simulink model "FaultSimulator"  (R2022a)
settings: Solver=FixedStepAuto  StartTime=0.0  StopTime=10.0  FixedStep=1e-3  RelTol=1e-3
values from neighbouring files (symbols this model uses):
  A = A_set(i)    [DatacreatorForChapter3.m]    (also in parameterSetting.m = 0.0005)
  base = Mb*9.8/4+mw*9.8+0.5*9.8*Mv    (= 46305)    [parameterSetting.m]
  d = 2.6    [parameterSetting.m]
  I = 3055e-8    (= 3.055e-05)    [parameterSetting.m]
  Jv = 3044    [parameterSetting.m]
  kf = 6.67e7    (= 66700000)    [parameterSetting.m]
  Kv = 1.5e6    (= 1500000)    [parameterSetting.m]
  L = beamLength(k)*210    [BuildDataSample.m]    (also in Figure3_1.m = 210; parameterSetting.m = 210)
  Ln = length_set(i)    [DatacreatorForChapter3.m]
  Ln2 = 0    [DatacreatorForChapter3.m]
  m = 7800*(7684e-6)    (= 59.9352)    [parameterSetting.m]
  Mv = 4060/2    (= 2030)    [parameterSetting.m]
  mw = 1670/2    (= 835)    [parameterSetting.m]
  N1 = 0    [DatacreatorForChapter3.m]
  N2 = 0    [DatacreatorForChapter3.m]
  position = 0.55*L    [DatacreatorForChapter3.m]    (also in Figure3_3ToFigure3_8andTable4_1.m = 115.5; Figure3_8.m = 115.5; Figure3_9.m = 105; parameterSetting.m = 105)
  r = 0.25    [Figure3_3ToFigure3_8andTable4_1.m]    (also in parameterSetting.m = 0.42)
  R = 0.42    [parameterSetting.m]
  v = vkmh/3.6    [BuildDataSample.m]    (also in DatacreatorForChapter3.m = vkmh_set(j)/3.6; Figure3_1.m = vkmh_set(i)/3.6; Figure3_3ToFigure3_8andTable4_1.m = 13.8888888889; Figure3_8.m = 50)
  vkmh = vkmh_set(j)    [BuildDataSample.m]    (also in parameterSetting.m = 100)
(part 1 of 157)
settings Model: Creator=Spicydel  LastModifiedBy=Spicydel

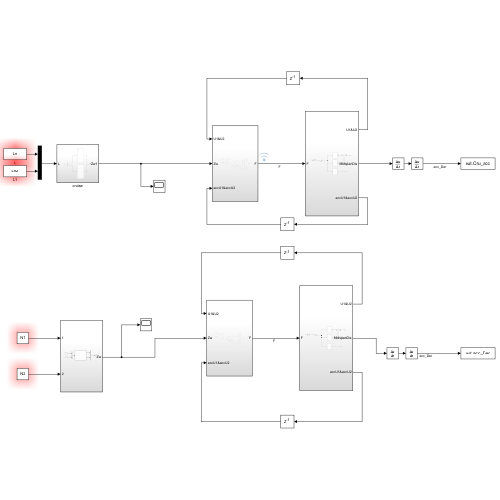
[thumbnail: Simulink's stored preview of the top-level diagram]
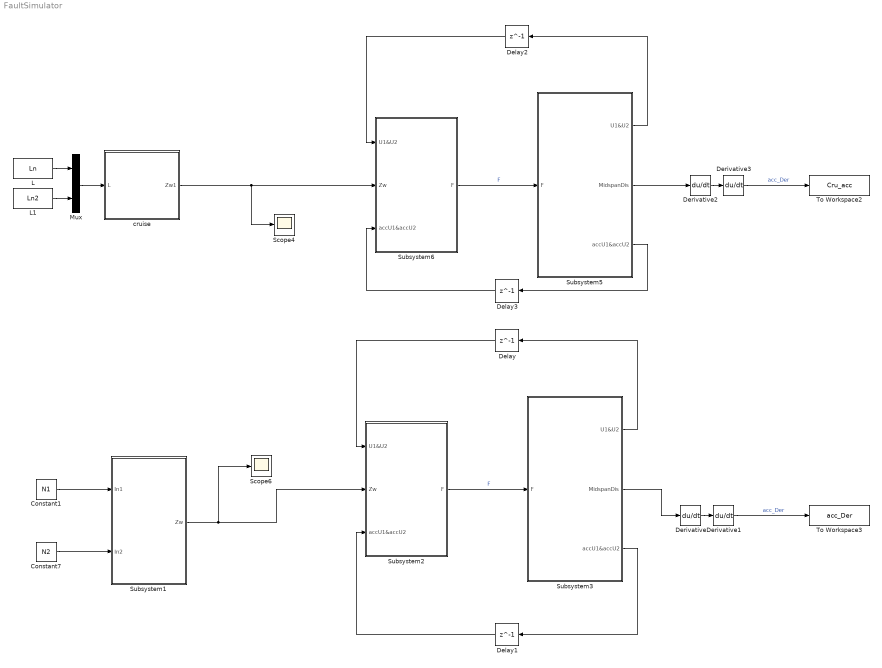
== system FaultSimulator ==
  Constant1 : Constant  Value=N1
  Constant7 : Constant  Value=N2
  Delay{,1..3} (4 alike) : Delay  DelayLength=1
  Derivative{,1..3} (4 alike) : Derivative
  L : Constant  Value=Ln
  L1 : Constant  Value=Ln2
  Mux : Mux  Inputs=2
  Scope4 : Scope
  Scope6 : Scope
  Subsystem1 : Subsystem
  Subsystem2 : Subsystem
  Subsystem3 : Subsystem
  Subsystem5 : Subsystem
  Subsystem6 : Subsystem
  To Workspace2 : ToWorkspace  VariableName=Cru_acc  SaveFormat=Timeseries  SampleTime=-1
  To Workspace3 : ToWorkspace  VariableName=acc_Der  SaveFormat=Timeseries  SampleTime=-1
  cruise : Subsystem
  wires:
  Subsystem5/2(MidspanDis) -> Derivative2/1
  Delay2/1 -> Subsystem6/1(U1&U2)
  Subsystem5/1(U1&U2) -> Delay2/1
  L/1 -> Mux/1
  Delay3/1 -> Subsystem6/3(accU1&accU2)
  Subsystem6/1(F) -> Subsystem5/1(F)  "F"
  cruise/1(Zw1) -> Scope4/1, Subsystem6/2(Zw)
  Derivative3/1 -> To Workspace2/1  "acc_Der"
  Derivative2/1 -> Derivative3/1
  Subsystem5/3(accU1&accU2) -> Delay3/1
  L1/1 -> Mux/2
  Mux/1 -> cruise/1(L)
  Subsystem3/1(U1&U2) -> Delay/1
  Derivative1/1 -> To Workspace3/1  "acc_Der"
  Constant1/1 -> Subsystem1/1(In1)
  Constant7/1 -> Subsystem1/2(In2)
  Subsystem3/2(MidspanDis) -> Derivative/1
  Delay/1 -> Subsystem2/1(U1&U2)
  Subsystem1/1(Zw) -> Scope6/1, Subsystem2/2(Zw)
  Subsystem2/1(F) -> Subsystem3/1(F)  "F"
  Subsystem3/3(accU1&accU2) -> Delay1/1
  Delay1/1 -> Subsystem2/3(accU1&accU2)
  Derivative/1 -> Derivative1/1

== system FaultSimulator/Subsystem1 ==
  In1 : Inport
  In2 : Inport  Port=2
  Mux1 : Mux  Inputs=2
  Mux2 : Mux  Inputs=2
  poly : Subsystem
  Zw : Outport
  wires:
  poly/1(wheel1) -> Mux2/1
  In2/1 -> Mux1/2
  poly/2(wheel2) -> Mux2/2
  Mux1/1 -> poly/1(N)
  In1/1 -> Mux1/1
  Mux2/1 -> Zw/1

== system FaultSimulator/Subsystem2 ==
  U1&U2 : Inport
  Zw : Inport  Port=2
  accU1&accU2 : Inport  Port=3
  Add : Sum  Inputs=+---
  Add1 : Sum  Inputs=-+
  Add2 : Sum  Inputs=--++-
  Add3 : Sum  Inputs=-++--
  Add4 : Sum
  Add5 : Sum  Inputs=-+
  Add6 : Sum  Inputs=-+
  Add7 : Sum  Inputs=++--
  Constant : Constant  Value=base
  Demux{,1..6} (7 alike) : Demux  Outputs=2
  Derivative{,1..3} (4 alike) : Derivative
  Gain : Gain  Gain=2*Kv/Mv
  Gain1 : Gain  Gain=Kv*d*d/Jv/2
  Gain12 : Gain  Gain=Kv/Mv
  Gain14 : Gain  Gain=Kv*d/Jv/2
  Gain2 : Gain  Gain=Kv
  Gain3 : Gain  Gain=Kv/Mv
  Gain4 : Gain  Gain=Kv*d/Jv/2
  Gain5 : Gain  Gain=d*Kv/2
  Gain7 : Gain  Gain=mw
  Gain8 : Gain  Gain=mw
  Gain9 : Gain  Gain=Kv
  Integrator{,1..3} (4 alike) : Integrator
  Mux : Mux  Inputs=2
  F : Outport
  wires:
  Integrator/1 -> Integrator1/1
  Integrator1/1 -> Gain2/1, Gain/1  "Zv"
  Add2/1 -> Integrator/1  "Z_dot_dot"
  Integrator2/1 -> Integrator3/1
  Integrator3/1 -> Gain5/1, Gain1/1  "theta"
  Add3/1 -> Integrator2/1  "theta_dot_dot"
  Gain5/1 -> Add/2, Add7/2
  Zw/1 -> [Zw]
  Gain/1 -> Add2/5  "Z_dot_dot"
  Demux/1 -> Add2/3
  Demux/2 -> Add2/4
  Gain1/1 -> Add3/5  "theta_dot_dot"
  Demux2/1 -> Add3/1
  Demux2/2 -> Add3/2
  U1&U2/1 -> [U1u2]
  Add4/1 -> F/1
  Constant/1 -> Add4/2
  [U1u2] -> Gain12/1
  Gain12/1 -> Demux1/1
  Demux1/2 -> Add2/2
  Demux1/1 -> Add2/1
  [U1u2] -> Gain14/1
  Gain14/1 -> Demux3/1
  Demux3/1 -> Add3/3
  Demux3/2 -> Add3/4
  Gain2/1 -> Add7/1, Add/1
  Mux/1 -> Add4/1
  [Zw] -> Gain3/1
  Gain3/1 -> Demux/1
  Gain4/1 -> Demux2/1
  [Zw] -> Gain4/1
  [U1u2] -> Add1/1
  [Zw] -> Demux6/1, Add1/2
  Add1/1 -> Gain9/1
  Derivative/1 -> Derivative1/1
  accU1&accU2/1 -> Demux5/1
  Demux6/1 -> Derivative/1
  Derivative2/1 -> Derivative3/1
  Demux6/2 -> Derivative2/1
  Derivative1/1 -> Add5/2
  Demux5/1 -> Add5/1
  Derivative3/1 -> Add6/2
  Demux5/2 -> Add6/1
  Demux4/1 -> Add/3
  Add5/1 -> Gain7/1
  Add6/1 -> Gain8/1
  Gain7/1 -> Add/4
  Gain9/1 -> Demux4/1
  Demux4/2 -> Add7/3
  Gain8/1 -> Add7/4
  Add7/1 -> Mux/2  "F2"
  Add/1 -> Mux/1  "F1"

== system FaultSimulator/Subsystem3 ==
  F : Inport
  Add : Sum
  Demux1 : Demux  Outputs=2
  Demux2 : Demux  Outputs=2
  Demux3 : Demux  Outputs=2
  Gain1 : Gain  Gain=-1
  Gain2 : Gain  Gain=-1
  Mux : Mux  Inputs=2
  Mux1 : Mux  Inputs=2
  Mux2 : Mux  Inputs=2
  Subsystem : Subsystem
  Subsystem1 : Subsystem
  Subsystem3 : Subsystem
  U1&U2 : Outport
  MidspanDis : Outport  Port=2
  accU1&accU2 : Outport  Port=3
  wires:
  Subsystem/1(u1) -> Gain1/1
  Subsystem1/1(u2) -> Gain2/1
  Subsystem3/1(Midspan) -> MidspanDis/1
  Gain1/1 -> Demux2/1
  F/1 -> Demux1/1
  Demux1/1 -> Add/1
  Demux1/2 -> Add/2
  Add/1 -> Mux1/2, Mux1/1
  Mux1/1 -> Subsystem3/1(F), Subsystem1/1(F), Subsystem/1(F)
  Gain2/1 -> Demux3/1
  Demux2/1 -> Mux/1
  Demux3/1 -> Mux/2
  Mux2/1 -> accU1&accU2/1
  Mux/1 -> U1&U2/1
  Demux2/2 -> Mux2/1
  Demux3/2 -> Mux2/2

== system FaultSimulator/Subsystem5 ==
  F : Inport
  Add : Sum
  Demux1 : Demux  Outputs=2
  Demux2 : Demux  Outputs=2
  Demux3 : Demux  Outputs=2
  Gain1 : Gain  Gain=-1
  Gain2 : Gain  Gain=-1
  Mux : Mux  Inputs=2
  Mux1 : Mux  Inputs=2
  Mux2 : Mux  Inputs=2
  Subsystem : Subsystem
  Subsystem1 : Subsystem
  Subsystem3 : Subsystem
  U1&U2 : Outport
  MidspanDis : Outport  Port=2
  accU1&accU2 : Outport  Port=3
  wires:
  Demux3/2 -> Mux2/2
  Demux2/2 -> Mux2/1
  Mux/1 -> U1&U2/1
  Mux2/1 -> accU1&accU2/1
  Demux3/1 -> Mux/2
  Demux2/1 -> Mux/1
  Gain2/1 -> Demux3/1
  Mux1/1 -> Subsystem/1(F), Subsystem1/1(F), Subsystem3/1(F)
  Add/1 -> Mux1/1, Mux1/2
  Demux1/2 -> Add/2
  Demux1/1 -> Add/1
  F/1 -> Demux1/1
  Gain1/1 -> Demux2/1
  Subsystem1/1(u2) -> Gain2/1
  Subsystem/1(u1) -> Gain1/1
  Subsystem3/1(Midspan) -> MidspanDis/1

== system FaultSimulator/Subsystem6 ==
  U1&U2 : Inport
  Zw : Inport  Port=2
  accU1&accU2 : Inport  Port=3
  Add : Sum  Inputs=+---
  Add1 : Sum  Inputs=-+
  Add2 : Sum  Inputs=--++-
  Add3 : Sum  Inputs=-++--
  Add4 : Sum
  Add5 : Sum  Inputs=-+
  Add6 : Sum  Inputs=-+
  Add7 : Sum  Inputs=++--
  Constant : Constant  Value=base
  Demux{,1..6} (7 alike) : Demux  Outputs=2
  Derivative{,1..3} (4 alike) : Derivative
  Gain : Gain  Gain=2*Kv/Mv
  Gain1 : Gain  Gain=Kv*d*d/Jv/2
  Gain12 : Gain  Gain=Kv/Mv
  Gain14 : Gain  Gain=Kv*d/Jv/2
  Gain2 : Gain  Gain=Kv
  Gain3 : Gain  Gain=Kv/Mv
  Gain4 : Gain  Gain=Kv*d/Jv/2
  Gain5 : Gain  Gain=d*Kv/2
  Gain7 : Gain  Gain=mw
  Gain8 : Gain  Gain=mw
  Gain9 : Gain  Gain=Kv
  Integrator{,1..3} (4 alike) : Integrator
  Mux : Mux  Inputs=2
  Scope : Scope
  Scope1 : Scope
  Scope2 : Scope
  F : Outport
  wires:
  Add/1 -> Scope1/1, Mux/1  "F1"
  Add7/1 -> Mux/2, Scope2/1  "F2"
  Gain8/1 -> Add7/4
  Demux4/2 -> Add7/3
  Gain9/1 -> Demux4/1
  Gain7/1 -> Add/4
  Add6/1 -> Gain8/1
  Add5/1 -> Gain7/1
  Demux4/1 -> Add/3
  Demux5/2 -> Add6/1
  Derivative3/1 -> Add6/2
  Demux5/1 -> Add5/1
  Derivative1/1 -> Add5/2
  Demux6/2 -> Derivative2/1
  Derivative2/1 -> Derivative3/1
  Demux6/1 -> Derivative/1
  accU1&accU2/1 -> Demux5/1
  Derivative/1 -> Derivative1/1
  Add1/1 -> Gain9/1
  [Zw] -> Add1/2, Demux6/1
  [U1u2] -> Add1/1
  [Zw] -> Gain4/1
  Gain4/1 -> Demux2/1
  Gain3/1 -> Demux/1
  [Zw] -> Gain3/1
  Mux/1 -> Add4/1
  Gain2/1 -> Add/1, Add7/1
  Demux3/2 -> Add3/4
  Demux3/1 -> Add3/3
  Gain14/1 -> Demux3/1
  [U1u2] -> Gain14/1
  Demux1/1 -> Add2/1
  Demux1/2 -> Add2/2
  Gain12/1 -> Demux1/1
  [U1u2] -> Gain12/1
  Constant/1 -> Add4/2
  Add4/1 -> Scope/1, F/1
  U1&U2/1 -> [U1u2]
  Demux2/2 -> Add3/2
  Demux2/1 -> Add3/1
  Gain1/1 -> Add3/5  "theta_dot_dot"
  Demux/2 -> Add2/4
  Demux/1 -> Add2/3
  Gain/1 -> Add2/5  "Z_dot_dot"
  Zw/1 -> [Zw]
  Gain5/1 -> Add7/2, Add/2
  Add3/1 -> Integrator2/1  "theta_dot_dot"
  Integrator3/1 -> Gain1/1, Gain5/1  "theta"
  Integrator2/1 -> Integrator3/1
  Add2/1 -> Integrator/1  "Z_dot_dot"
  Integrator1/1 -> Gain/1, Gain2/1  "Zv"
  Integrator/1 -> Integrator1/1

== system FaultSimulator/cruise ==
  L : Inport
  Demux : Demux  Outputs=2
  Mux : Mux  Inputs=2
  Subsystem1 : Subsystem
  Subsystem2 : Subsystem
  Zw1 : Outport
  wires:
  L/1 -> Demux/1
  Demux/1 -> Subsystem1/1(In1)
  Demux/2 -> Subsystem2/1(In1)
  Subsystem1/1(Output) -> Mux/1
  Subsystem2/1(Output) -> Mux/2
  Mux/1 -> Zw1/1

== system FaultSimulator/Subsystem1/poly ==
  N : Inport
  Clock : Clock
  Constant : Constant  Value=v
  Demux : Demux  Outputs=2
  Gain1 : Gain  Gain=1/r
  Product1 : Product
  Subsystem : Subsystem
  Subsystem1 : Subsystem
  wheel1 : Outport
  wheel2 : Outport  Port=2
  wires:
  Constant/1 -> Gain1/1
  Gain1/1 -> Product1/1
  Clock/1 -> Product1/2
  Product1/1 -> [omega]
  [omega] -> Subsystem/2(In2)
  Subsystem/1(Out1) -> wheel1/1
  [omega] -> Subsystem1/2(In2)
  Subsystem1/1(Out1) -> wheel2/1
  N/1 -> Demux/1
  Demux/1 -> Subsystem/1(In1)
  Demux/2 -> Subsystem1/1(In1)

== system FaultSimulator/Subsystem3/Subsystem ==
  F : Inport
  Add : Sum  Inputs=+++++++
  Constant1 : Constant  Value=21
  Constant11 : Constant
  Constant2 : Constant  Value=41
  Constant3 : Constant  Value=61
  Constant4 : Constant  Value=81
  Constant5 : Constant  Value=101
  Constant6 : Constant  Value=121
  Subsystem : Subsystem
  Subsystem1 : Subsystem
  Subsystem2 : Subsystem
  Subsystem3 : Subsystem
  Subsystem4 : Subsystem
  Subsystem5 : Subsystem
  Subsystem6 : Subsystem
  u1 : Outport
  wires:
  F/1 -> [Ff]
  Constant11/1 -> Subsystem/1(In1)
  [Ff] -> Subsystem/2(In2)
  [Ff] -> Subsystem1/2(In2)
  Constant1/1 -> Subsystem1/1(In1)
  Constant2/1 -> Subsystem2/1(In1)
  [Ff] -> Subsystem2/2(In2)
  [Ff] -> Subsystem3/2(In2)
  Constant3/1 -> Subsystem3/1(In1)
  Constant4/1 -> Subsystem4/1(In1)
  [Ff] -> Subsystem4/2(In2)
  Subsystem/1(Out1) -> Add/1
  Subsystem1/1(Out1) -> Add/2
  Subsystem2/1(Out1) -> Add/3
  Subsystem3/1(Out1) -> Add/4
  Subsystem4/1(Out1) -> Add/5
  Add/1 -> u1/1
  [Ff] -> Subsystem5/2(In2)
  Constant5/1 -> Subsystem5/1(In1)
  Subsystem5/1(Out1) -> Add/6
  Constant6/1 -> Subsystem6/1(In1)
  [Ff] -> Subsystem6/2(In2)
  Subsystem6/1(Out1) -> Add/7

== system FaultSimulator/Subsystem3/Subsystem1 ==
  F : Inport
  Add : Sum  Inputs=+++++++
  Constant1 : Constant  Value=21
  Constant11 : Constant
  Constant2 : Constant  Value=61
  Constant3 : Constant  Value=41
  Constant4 : Constant  Value=101
  Constant5 : Constant  Value=81
  Constant6 : Constant  Value=121
  Subsystem : Subsystem
  Subsystem1 : Subsystem
  Subsystem2 : Subsystem
  Subsystem3 : Subsystem
  Subsystem4 : Subsystem
  Subsystem5 : Subsystem
  Subsystem6 : Subsystem
  u2 : Outport
  wires:
  F/1 -> [Ff]
  Constant11/1 -> Subsystem/1(In1)
  Subsystem/1(Out1) -> Add/1
  [Ff] -> Subsystem/2(In2)
  [Ff] -> Subsystem1/2(In2)
  Constant1/1 -> Subsystem1/1(In1)
  Constant2/1 -> Subsystem3/1(In1)
  [Ff] -> Subsystem3/2(In2)
  [Ff] -> Subsystem2/2(In2)
  Constant3/1 -> Subsystem2/1(In1)
  Constant5/1 -> Subsystem4/1(In1)
  [Ff] -> Subsystem4/2(In2)
  Subsystem1/1(Out1) -> Add/2
  Subsystem2/1(Out1) -> Add/3
  Subsystem3/1(Out1) -> Add/4
  Subsystem4/1(Out1) -> Add/5
  Add/1 -> u2/1
  [Ff] -> Subsystem6/2(In2)
  Constant6/1 -> Subsystem6/1(In1)
  [Ff] -> Subsystem5/2(In2)
  Constant4/1 -> Subsystem5/1(In1)
  Subsystem5/1(Out1) -> Add/6
  Subsystem6/1(Out1) -> Add/7

== system FaultSimulator/Subsystem3/Subsystem3 ==
  F : Inport
  Add : Sum  Inputs=+++++++
  Constant1 : Constant  Value=21
  Constant11 : Constant
  Constant2 : Constant  Value=41
  Constant3 : Constant  Value=61
  Constant4 : Constant  Value=81
  Constant5 : Constant  Value=101
  Constant6 : Constant  Value=121
  Subsystem1 : Subsystem
  Subsystem2 : Subsystem
  Subsystem3 : Subsystem
  Subsystem4 : Subsystem
  Subsystem5 : Subsystem
  Subsystem6 : Subsystem
  Subsystem7 : Subsystem
  Midspan : Outport
  wires:
  F/1 -> [Ff]
  Constant11/1 -> Subsystem5/2(In1)
  Subsystem5/1(Out1) -> Add/1
  Constant1/1 -> Subsystem1/2(In1)
  Subsystem1/1(Out1) -> Add/2
  Constant2/1 -> Subsystem2/2(In1)
  Subsystem2/1(Out1) -> Add/3
  Add/1 -> Midspan/1
  [Ff] -> Subsystem5/1(In2)
  [Ff] -> Subsystem1/1(In2)
  [Ff] -> Subsystem2/1(In2)
  [Ff] -> Subsystem3/1(In2)
  Constant3/1 -> Subsystem3/2(In1)
  Constant4/1 -> Subsystem4/2(In1)
  [Ff] -> Subsystem4/1(In2)
  Subsystem3/1(Out1) -> Add/4
  Subsystem4/1(Out1) -> Add/5
  [Ff] -> Subsystem6/1(In2)
  Constant5/1 -> Subsystem6/2(In1)
  Constant6/1 -> Subsystem7/2(In1)
  [Ff] -> Subsystem7/1(In2)
  Subsystem6/1(Out1) -> Add/6
  Subsystem7/1(Out1) -> Add/7

== system FaultSimulator/Subsystem5/Subsystem ==
  F : Inport
  Add : Sum  Inputs=+++++++
  Constant1 : Constant  Value=21
  Constant11 : Constant
  Constant2 : Constant  Value=41
  Constant3 : Constant  Value=61
  Constant4 : Constant  Value=81
  Constant5 : Constant  Value=101
  Constant6 : Constant  Value=121
  Subsystem : Subsystem
  Subsystem1 : Subsystem
  Subsystem2 : Subsystem
  Subsystem3 : Subsystem
  Subsystem4 : Subsystem
  Subsystem5 : Subsystem
  Subsystem6 : Subsystem
  u1 : Outport
  wires:
  Subsystem6/1(Out1) -> Add/7
  [Ff] -> Subsystem6/2(In2)
  Constant6/1 -> Subsystem6/1(In1)
  Subsystem5/1(Out1) -> Add/6
  Constant5/1 -> Subsystem5/1(In1)
  [Ff] -> Subsystem5/2(In2)
  Add/1 -> u1/1
  Subsystem4/1(Out1) -> Add/5
  Subsystem3/1(Out1) -> Add/4
  Subsystem2/1(Out1) -> Add/3
  Subsystem1/1(Out1) -> Add/2
  Subsystem/1(Out1) -> Add/1
  [Ff] -> Subsystem4/2(In2)
  Constant4/1 -> Subsystem4/1(In1)
  Constant3/1 -> Subsystem3/1(In1)
  [Ff] -> Subsystem3/2(In2)
  [Ff] -> Subsystem2/2(In2)
  Constant2/1 -> Subsystem2/1(In1)
  Constant1/1 -> Subsystem1/1(In1)
  [Ff] -> Subsystem1/2(In2)
  [Ff] -> Subsystem/2(In2)
  Constant11/1 -> Subsystem/1(In1)
  F/1 -> [Ff]

== system FaultSimulator/Subsystem5/Subsystem1 ==
  F : Inport
  Add : Sum  Inputs=+++++++
  Constant1 : Constant  Value=21
  Constant11 : Constant
  Constant2 : Constant  Value=61
  Constant3 : Constant  Value=41
  Constant4 : Constant  Value=101
  Constant5 : Constant  Value=81
  Constant6 : Constant  Value=121
  Subsystem : Subsystem
  Subsystem1 : Subsystem
  Subsystem2 : Subsystem
  Subsystem3 : Subsystem
  Subsystem4 : Subsystem
  Subsystem5 : Subsystem
  Subsystem6 : Subsystem
  u2 : Outport
  wires:
  Subsystem6/1(Out1) -> Add/7
  Subsystem5/1(Out1) -> Add/6
  Constant4/1 -> Subsystem5/1(In1)
  [Ff] -> Subsystem5/2(In2)
  Constant6/1 -> Subsystem6/1(In1)
  [Ff] -> Subsystem6/2(In2)
  Add/1 -> u2/1
  Subsystem4/1(Out1) -> Add/5
  Subsystem3/1(Out1) -> Add/4
  Subsystem2/1(Out1) -> Add/3
  Subsystem1/1(Out1) -> Add/2
  [Ff] -> Subsystem4/2(In2)
  Constant5/1 -> Subsystem4/1(In1)
  Constant3/1 -> Subsystem2/1(In1)
  [Ff] -> Subsystem2/2(In2)
  [Ff] -> Subsystem3/2(In2)
  Constant2/1 -> Subsystem3/1(In1)
  Constant1/1 -> Subsystem1/1(In1)
  [Ff] -> Subsystem1/2(In2)
  [Ff] -> Subsystem/2(In2)
  Subsystem/1(Out1) -> Add/1
  Constant11/1 -> Subsystem/1(In1)
  F/1 -> [Ff]

== system FaultSimulator/Subsystem5/Subsystem3 ==
  F : Inport
  Add : Sum  Inputs=+++++++
  Constant1 : Constant  Value=21
  Constant11 : Constant
  Constant2 : Constant  Value=41
  Constant3 : Constant  Value=61
  Constant4 : Constant  Value=81
  Constant5 : Constant  Value=101
  Constant6 : Constant  Value=121
  Subsystem1 : Subsystem
  Subsystem2 : Subsystem
  Subsystem3 : Subsystem
  Subsystem4 : Subsystem
  Subsystem5 : Subsystem
  Subsystem6 : Subsystem
  Subsystem7 : Subsystem
  Midspan : Outport
  wires:
  Subsystem7/1(Out1) -> Add/7
  Subsystem6/1(Out1) -> Add/6
  [Ff] -> Subsystem7/1(In2)
  Constant6/1 -> Subsystem7/2(In1)
  Constant5/1 -> Subsystem6/2(In1)
  [Ff] -> Subsystem6/1(In2)
  Subsystem4/1(Out1) -> Add/5
  Subsystem3/1(Out1) -> Add/4
  [Ff] -> Subsystem4/1(In2)
  Constant4/1 -> Subsystem4/2(In1)
  Constant3/1 -> Subsystem3/2(In1)
  [Ff] -> Subsystem3/1(In2)
  [Ff] -> Subsystem2/1(In2)
  [Ff] -> Subsystem1/1(In2)
  [Ff] -> Subsystem5/1(In2)
  Add/1 -> Midspan/1
  Subsystem2/1(Out1) -> Add/3
  Constant2/1 -> Subsystem2/2(In1)
  Subsystem1/1(Out1) -> Add/2
  Constant1/1 -> Subsystem1/2(In1)
  Subsystem5/1(Out1) -> Add/1
  Constant11/1 -> Subsystem5/2(In1)
  F/1 -> [Ff]

== system FaultSimulator/cruise/Subsystem1 ==
  In1 : Inport
  Constant : Constant  Value=0
  Gain8 : Gain  Gain=2/pi
  Subsystem : Subsystem
  Switch : Switch  Criteria=u2 ~= 0  SaturateOnIntegerOverflow=off  InputSameDT=off
  Output : Outport
  wires:
  Gain8/1 -> Subsystem/2(L0)
  In1/1 -> Subsystem/1(L), Switch/2, Gain8/1
  Switch/1 -> Output/1
  Constant/1 -> Switch/3
  Subsystem/1(Output) -> Switch/1

== system FaultSimulator/cruise/Subsystem2 ==
  In1 : Inport
  Constant : Constant  Value=0
  Gain8 : Gain  Gain=2/pi
  Subsystem : Subsystem
  Switch : Switch  Criteria=u2 ~= 0  SaturateOnIntegerOverflow=off  InputSameDT=off
  Output : Outport
  wires:
  Constant/1 -> Switch/3
  Subsystem/1(Output) -> Switch/1
  Switch/1 -> Output/1
  In1/1 -> Gain8/1, Switch/2, Subsystem/1(L)
  Gain8/1 -> Subsystem/2(L0)

== system FaultSimulator/Subsystem1/poly/Subsystem ==
  In1 : Inport
  In2 : Inport  Port=2
  Add : Sum
  Add1 : Sum
  Add2 : Sum  Inputs=+-
  Constant : Constant  Value=r
  Constant1 : Constant  Value=r
  Constant2 : Constant  Value=0
  Cos : Trigonometry  Operator=cos
  Derivative : Derivative
  Derivative1 : Derivative
  Gain2 : Gain  Gain=A
  Product : Product
  Product2 : Product
  Product3 : Product
  Scope : Scope
  Sin : Trigonometry
  Sin1 : Trigonometry
  Sqrt : Sqrt
  Square : Math  Operator=square
  Square1 : Math  Operator=square
  Switch : Switch  Criteria=u2 ~= 0  SaturateOnIntegerOverflow=off  InputSameDT=off
  Out1 : Outport
  wires:
  Cos/1 -> Product3/2
  Product/1 -> Sin/1
  Sin/1 -> Gain2/1
  Gain2/1 -> Add/1
  Add/1 -> Product3/1, Product2/2
  Constant/1 -> Add/2
  [omega] -> Sin1/1
  Sin1/1 -> Product2/1
  [omega] -> Cos/1
  Product2/1 -> Square/1
  Constant1/1 -> Add2/2
  Sqrt/1 -> Add2/1
  Square1/1 -> Add1/2
  Product3/1 -> Square1/1
  Square/1 -> Add1/1
  Add1/1 -> Sqrt/1
  In2/1 -> [omega], Product/2
  [N] -> Product/1
  In1/1 -> [N]
  [N] -> Switch/2
  Switch/1 -> Derivative/1, Out1/1  "Rt_poly"
  Add2/1 -> Switch/1
  Constant2/1 -> Switch/3
  Derivative/1 -> Derivative1/1
  Derivative1/1 -> Scope/1

== system FaultSimulator/Subsystem1/poly/Subsystem1 ==
  In1 : Inport
  In2 : Inport  Port=2
  Add : Sum
  Add1 : Sum
  Add2 : Sum  Inputs=+-
  Constant : Constant  Value=r
  Constant1 : Constant  Value=r
  Constant2 : Constant  Value=0
  Cos : Trigonometry  Operator=cos
  Gain2 : Gain  Gain=A
  Product : Product
  Product2 : Product
  Product3 : Product
  Sin : Trigonometry
  Sin1 : Trigonometry
  Sqrt : Sqrt
  Square : Math  Operator=square
  Square1 : Math  Operator=square
  Switch : Switch  Criteria=u2 ~= 0  SaturateOnIntegerOverflow=off  InputSameDT=off
  Out1 : Outport
  wires:
  Constant2/1 -> Switch/3
  Add2/1 -> Switch/1
  Switch/1 -> Out1/1
  [N] -> Switch/2
  In1/1 -> [N]
  [N] -> Product/1
  In2/1 -> Product/2, [omega]
  Add1/1 -> Sqrt/1
  Square/1 -> Add1/1
  Product3/1 -> Square1/1
  Square1/1 -> Add1/2
  Sqrt/1 -> Add2/1
  Constant1/1 -> Add2/2
  Product2/1 -> Square/1
  [omega] -> Cos/1
  Sin1/1 -> Product2/1
  [omega] -> Sin1/1
  Constant/1 -> Add/2
  Add/1 -> Product2/2, Product3/1
  Gain2/1 -> Add/1
  Sin/1 -> Gain2/1
  Product/1 -> Sin/1
  Cos/1 -> Product3/2

== system FaultSimulator/Subsystem3/Subsystem/Subsystem ==
  In1 : Inport
  In2 : Inport  Port=2
  Add : Sum  Inputs=++++++++++++++++++++
  Add Constant{,1..18} (19 alike) : Bias  Bias=1  SaturateOnIntegerOverflow=off
  Subsystem1 : Subsystem
  Subsystem10 : Subsystem
  Subsystem11 : Subsystem
  Subsystem12 : Subsystem
  Subsystem13 : Subsystem
  Subsystem14 : Subsystem
  Subsystem15 : Subsystem
  Subsystem16 : Subsystem
  Subsystem17 : Subsystem
  Subsystem18 : Subsystem
  Subsystem19 : Subsystem
  Subsystem2 : Subsystem
  Subsystem20 : Subsystem
  Subsystem3 : Subsystem
  Subsystem4 : Subsystem
  Subsystem5 : Subsystem
  Subsystem6 : Subsystem
  Subsystem7 : Subsystem
  Subsystem8 : Subsystem
  Subsystem9 : Subsystem
  Out1 : Outport
  wires:
  Subsystem14/1(Out1) -> Add/9
  Add Constant9/1 -> Subsystem11/1(index), Add Constant10/1
  Add Constant2/1 -> Subsystem2/1(index), Add Constant3/1
  [Ff] -> Subsystem13/2(F)
  Add Constant11/1 -> Subsystem16/1(index), Add Constant12/1
  Add Constant14/1 -> Subsystem13/1(index), Add Constant15/1
  Subsystem8/1(Out1) -> Add/5
  [Ff] -> Subsystem17/2(F)
  Add Constant15/1 -> Subsystem20/1(index), Add Constant16/1
  Subsystem1/1(Out1) -> Add/2
  Add Constant/1 -> Subsystem1/1(index), Add Constant1/1
  Add Constant3/1 -> Subsystem8/1(index), Add Constant4/1
  Subsystem9/1(Out1) -> Add/10
  [Ff] -> Subsystem3/2(F)
  Add Constant1/1 -> Subsystem3/1(index), Add Constant2/1
  Subsystem7/1(Out1) -> Add/7
  Add Constant17/1 -> Subsystem19/1(index), Add Constant18/1
  Add Constant18/1 -> Subsystem18/1(index)
  [Ff] -> Subsystem15/2(F)
  [Ff] -> Subsystem10/2(F)
  Subsystem5/1(Out1) -> Add/8
  Subsystem10/1(Out1) -> Add/12
  Subsystem6/1(Out1) -> Add/1
  Add Constant16/1 -> Subsystem17/1(index), Add Constant17/1
  Add Constant10/1 -> Subsystem10/1(index), Add Constant11/1
  [Ff] -> Subsystem20/2(F)
  Subsystem17/1(Out1) -> Add/18
  Subsystem19/1(Out1) -> Add/19
  [Ff] -> Subsystem1/2(F)
  [Ff] -> Subsystem19/2(F)
  Add Constant8/1 -> Subsystem9/1(index), Add Constant9/1
  [Ff] -> Subsystem2/2(F)
  Subsystem3/1(Out1) -> Add/3
  [Ff] -> Subsystem8/2(F)
  Subsystem20/1(Out1) -> Add/17
  Subsystem12/1(Out1) -> Add/14
  In1/1 -> Subsystem6/1(index), Add Constant/1
  Subsystem16/1(Out1) -> Add/13
  Subsystem13/1(Out1) -> Add/16
  [Ff] -> Subsystem6/2(F)
  [Ff] -> Subsystem7/2(F)
  [Ff] -> Subsystem11/2(F)
  Subsystem15/1(Out1) -> Add/15
  Add Constant13/1 -> Subsystem15/1(index), Add Constant14/1
  Subsystem2/1(Out1) -> Add/4
  Add Constant12/1 -> Subsystem12/1(index), Add Constant13/1
  Subsystem4/1(Out1) -> Add/6
  [Ff] -> Subsystem4/2(F)
  [Ff] -> Subsystem9/2(F)
  [Ff] -> Subsystem5/2(F)
  [Ff] -> Subsystem14/2(F)
  [Ff] -> Subsystem12/2(F)
  Subsystem18/1(Out1) -> Add/20